simulink model "med_16a_2x_r113a"  (Simulink 7.4) (continued)
== system med_16a_2x_r113a/buffer1 == (continued)
      mask Help: eval('');xlDoc('-book','sysgen','-topic','Register');
      mask Display:
      | fprintf('','COMMENT: begin icon graphics');
      | patch([0 1 1 0 ],[0 0 1 1 ],[0.77 0.82 0.91]);
      | patch([0.25 0.1 0.316667 0.1 0.25 0.5 0.566667 0.633333 0.9 0.683333 0.483333 0.333333 0.533333 0.333333 0.483333 0.683333 0.9 0.633333 0.566667 0.5 0.25 ],[0.107143 0.267857 0.5 0.732143 0.892857 0.892857 0.821429 0.892857 0.892857 0.660714 0.875 0.714286 0.5 0.285714 0.125 0.339286 0.107143 0.107143 0.178571 0.107143 0.107143 ],[0.98 0.96 0.92]);
      | plot([0 1 1 0 0 ],[0 0 1 1 0 ]);
      | fprintf('','COMMENT: end icon graphics');
      | fprintf('','COMMENT: begin icon text');
      | color('black');port_label('input',1,'d');
      | color('black');port_label('input',2,'en');
      | color('black');port_label('output',1,'q');
      | color('black');disp('\bf{z^{-1}}','texmode','on');
      | fprintf('','COMMENT: end icon text');
  Register6 : S-Function  LoadFcn=xlBlockLoadCallback(gcbh);  FunctionName=sysgen  Parameters=ptable_  EnableBusSupport=off  mask(Xilinx Register Block){init=0, rst_sgadvanced=, rst=on, en_sgadvanced=, en=on, dbl_ovrd_sgadvanced=, dbl_ovrd=off, xl_use_area_sgadvanced=, xl_use_area=off, xl_area=[0,0,0,0,0,0,0], has_advanced_control=0, sggui_pos=21,47,436,219, block_type=register, block_version=11.4, sg_icon_stat=60,80,3,1,white,blue,0,b821322c,right,, sg_mask_display=|, sg_list_contents=, sg_blockgui_xml=}
      mask sg_mask_display=|
      | fprintf('','COMMENT: begin icon graphics');
      | patch([0 1 1 0 ],[0 0 1 1 ],[0.77 0.82 0.91]);
      | patch([0.233333 0.0666667 0.3 0.0666667 0.233333 0.5 0.566667 0.633333 0.916667 0.7 0.483333 0.333333 0.583333 0.333333 0.483333 0.7 0.916667 0.633333 0.566667 0.5 0.233333 ],[0.2 0.325 0.5 0.675 0.8 0.8 0.75 0.8 0.8 0.6375 0.8 0.6875 0.5 0.3125 0.2 0.3625 0.2 0.2 0.25 0.2 0.2 ],[0.98 0.96 0.92]);
      | plot([0 1 1 0 0 ],[0 0 1 1 0 ]);
      | fprintf('','COMMENT: end icon graphics');
      | fprintf('','COMMENT: begin icon text');
      | color('black');port_label('input',1,'d');
      | color('black');port_label('input',2,'rst');
      | color('black');port_label('input',3,'en');
      | color('black');port_label('output',1,'q');
      | color('black');disp('\bf{z^{-1}}','texmode','on');
      | fprintf('','COMMENT: end icon text');
      mask prompts: init "Initial value"; rst_sgadvanced ""; rst "Provide synchronous reset port"; en_sgadvanced ""; en "Provide enable port"; dbl_ovrd_sgadvanced ""; dbl_ovrd "Override with doubles"; xl_use_area_sgadvanced ""; xl_use_area "Define FPGA area for resource estimation"; xl_area "FPGA area [slices, FFs, BRAMs, LUTs, IOBs, emb. mults, TBUFs]"; has_advanced_control ""; sggui_pos ""; block_type ""; block_version ""; sg_icon_stat ""; sg_mask_display ""; sg_list_contents ""; sg_blockgui_xml ""
      mask help: Xilinx Register
      mask init: same as med_16a_2x_r113a/buffer1/Register2
      settings: CopyFcn=xlBlockMoveCallback(gcbh);  DeleteFcn=xlDestroyGui(gcbh);  ModelCloseFcn=xlDestroyGui(gcbh);  PreSaveFcn=xlBlockPreSaveCallback(gcbh);  PostSaveFcn=xlBlockPostSaveCallback(gcbh);  DestroyFcn=xlDestroyGui(gcbh);  OpenFcn=xlOpenGui(gcbh, 'register_gui.xml', @xlmagicenablement, -1)  CloseFcn=xlDestroyGui(gcbh);  MoveFcn=xlBlockMoveCallback(gcbh);
      mask Help: eval('');xlDoc('-book','sysgen','-topic','Register');
      mask Display:
      | fprintf('','COMMENT: begin icon graphics');
      | patch([0 1 1 0 ],[0 0 1 1 ],[0.77 0.82 0.91]);
      | patch([0.233333 0.0666667 0.3 0.0666667 0.233333 0.5 0.566667 0.633333 0.916667 0.7 0.483333 0.333333 0.583333 0.333333 0.483333 0.7 0.916667 0.633333 0.566667 0.5 0.233333 ],[0.2 0.325 0.5 0.675 0.8 0.8 0.75 0.8 0.8 0.6375 0.8 0.6875 0.5 0.3125 0.2 0.3625 0.2 0.2 0.25 0.2 0.2 ],[0.98 0.96 0.92]);
      | plot([0 1 1 0 0 ],[0 0 1 1 0 ]);
      | fprintf('','COMMENT: end icon graphics');
      | fprintf('','COMMENT: begin icon text');
      | color('black');port_label('input',1,'d');
      | color('black');port_label('input',2,'rst');
      | color('black');port_label('input',3,'en');
      | color('black');port_label('output',1,'q');
      | color('black');disp('\bf{z^{-1}}','texmode','on');
      | fprintf('','COMMENT: end icon text');
  Register7 : S-Function  LoadFcn=xlBlockLoadCallback(gcbh);  FunctionName=sysgen  Parameters=ptable_  EnableBusSupport=off  mask(Xilinx Register Block){init=0, rst_sgadvanced=, rst=on, en_sgadvanced=, en=on, dbl_ovrd_sgadvanced=, dbl_ovrd=off, xl_use_area_sgadvanced=, xl_use_area=off, xl_area=[0,0,0,0,0,0,0], has_advanced_control=0, sggui_pos=0,0,436,219, block_type=register, block_version=11.4, sg_icon_stat=60,74,3,1,white,blue,0,b821322c,right,, sg_mask_display=|, sg_list_contents=, sg_blockgui_xml=}
      mask sg_mask_display=|
      | fprintf('','COMMENT: begin icon graphics');
      | patch([0 1 1 0 ],[0 0 1 1 ],[0.77 0.82 0.91]);
      | patch([0.233333 0.0666667 0.3 0.0666667 0.233333 0.5 0.566667 0.633333 0.916667 0.7 0.483333 0.333333 0.583333 0.333333 0.483333 0.7 0.916667 0.633333 0.566667 0.5 0.233333 ],[0.175676 0.310811 0.5 0.689189 0.824324 0.824324 0.77027 0.824324 0.824324 0.648649 0.824324 0.702703 0.5 0.297297 0.175676 0.351351 0.175676 0.175676 0.22973 0.175676 0.175676 ],[0.98 0.96 0.92]);
      | plot([0 1 1 0 0 ],[0 0 1 1 0 ]);
      | fprintf('','COMMENT: end icon graphics');
      | fprintf('','COMMENT: begin icon text');
      | color('black');port_label('input',1,'d');
      | color('black');port_label('input',2,'rst');
      | color('black');port_label('input',3,'en');
      | color('black');port_label('output',1,'q');
      | color('black');disp('\bf{z^{-1}}','texmode','on');
      | fprintf('','COMMENT: end icon text');
      mask prompts: init "Initial value"; rst_sgadvanced ""; rst "Provide synchronous reset port"; en_sgadvanced ""; en "Provide enable port"; dbl_ovrd_sgadvanced ""; dbl_ovrd "Override with doubles"; xl_use_area_sgadvanced ""; xl_use_area "Define FPGA area for resource estimation"; xl_area "FPGA area [slices, FFs, BRAMs, LUTs, IOBs, emb. mults, TBUFs]"; has_advanced_control ""; sggui_pos ""; block_type ""; block_version ""; sg_icon_stat ""; sg_mask_display ""; sg_list_contents ""; sg_blockgui_xml ""
      mask help: Xilinx Register
      mask init: same as med_16a_2x_r113a/buffer1/Register2
      settings: CopyFcn=xlBlockMoveCallback(gcbh);  DeleteFcn=xlDestroyGui(gcbh);  ModelCloseFcn=xlDestroyGui(gcbh);  PreSaveFcn=xlBlockPreSaveCallback(gcbh);  PostSaveFcn=xlBlockPostSaveCallback(gcbh);  DestroyFcn=xlDestroyGui(gcbh);  OpenFcn=xlOpenGui(gcbh, 'register_gui.xml', @xlmagicenablement, -1)  CloseFcn=xlDestroyGui(gcbh);  MoveFcn=xlBlockMoveCallback(gcbh);
      mask Help: eval('');xlDoc('-book','sysgen','-topic','Register');
      mask Display:
      | fprintf('','COMMENT: begin icon graphics');
      | patch([0 1 1 0 ],[0 0 1 1 ],[0.77 0.82 0.91]);
      | patch([0.233333 0.0666667 0.3 0.0666667 0.233333 0.5 0.566667 0.633333 0.916667 0.7 0.483333 0.333333 0.583333 0.333333 0.483333 0.7 0.916667 0.633333 0.566667 0.5 0.233333 ],[0.175676 0.310811 0.5 0.689189 0.824324 0.824324 0.77027 0.824324 0.824324 0.648649 0.824324 0.702703 0.5 0.297297 0.175676 0.351351 0.175676 0.175676 0.22973 0.175676 0.175676 ],[0.98 0.96 0.92]);
      | plot([0 1 1 0 0 ],[0 0 1 1 0 ]);
      | fprintf('','COMMENT: end icon graphics');
      | fprintf('','COMMENT: begin icon text');
      | color('black');port_label('input',1,'d');
      | color('black');port_label('input',2,'rst');
      | color('black');port_label('input',3,'en');
      | color('black');port_label('output',1,'q');
      | color('black');disp('\bf{z^{-1}}','texmode','on');
      | fprintf('','COMMENT: end icon text');
  Relational1 : S-Function  LoadFcn=xlBlockLoadCallback(gcbh);  FunctionName=sysgen  Parameters=ptable_  EnableBusSupport=off  mask(Xilinx Arithmetic Relational Operator Block){mode_sgadvanced=, mode=a=b, en_sgadvanced=, en=off, latency=1, dbl_ovrd_sgadvanced=, dbl_ovrd=off, xl_use_area_sgadvanced=, xl_use_area=off, xl_area=[0, 0, 0, 0, 0, 0, 0], has_advanced_control=0, sggui_pos=21,72,436,219, block_type=relational, block_version=9.2.01, sg_icon_stat=55,51,2,1,white,blue,0,2d4703fd,right,, sg_mask_display=|, sg_list_contents={'table'=>{'mode'=>'popup(a=b|a!=b|a<b|a>b|a<=b|a>=b)','userSelections'=>{'mode'=>'a=b'}}}, sg_blockgui_xml=}
      mask sg_mask_display=|
      | fprintf('','COMMENT: begin icon graphics');
      | patch([0 1 1 0 ],[0 0 1 1 ],[0.77 0.82 0.91]);
      | patch([0.236364 0.0909091 0.309091 0.0909091 0.236364 0.472727 0.545455 0.618182 0.872727 0.672727 0.472727 0.327273 0.545455 0.327273 0.472727 0.672727 0.872727 0.618182 0.545455 0.472727 0.236364 ],[0.117647 0.27451 0.509804 0.745098 0.901961 0.901961 0.823529 0.901961 0.901961 0.686275 0.901961 0.745098 0.509804 0.27451 0.117647 0.333333 0.117647 0.117647 0.196078 0.117647 0.117647 ],[0.98 0.96 0.92]);
      | plot([0 1 1 0 0 ],[0 0 1 1 0 ]);
      | fprintf('','COMMENT: end icon graphics');
      | fprintf('','COMMENT: begin icon text');
      | color('black');port_label('input',1,'a');
      | color('black');port_label('input',2,'b');
      | color('black');disp('\newline\bf{a=b}\newlinez^{-1}','texmode','on');
      | fprintf('','COMMENT: end icon text');
      mask prompts: mode_sgadvanced ""; mode "Comparison:"; en_sgadvanced ""; en "Provide enable port"; dbl_ovrd_sgadvanced ""; dbl_ovrd "Override with doubles"; xl_use_area_sgadvanced ""; xl_use_area "Define FPGA area for resource estimation"; xl_area "FPGA area [slices, FFs, BRAMs, LUTs, IOBs, emb. mults, TBUFs]"; has_advanced_control ""; sggui_pos ""; block_type ""; block_version ""; sg_icon_stat ""; sg_mask_display ""; sg_list_contents ""; sg_blockgui_xml ""
      mask help: Xilinx Arithmetic Relational Operator
      mask init:
      | try
      |   tmp_gcb = gcb;
      |   tmp_gcbh = gcbh;
      |   if (strcmp('SysGenIndex',get_param(bdroot(tmp_gcbh),'tag')) && ~isempty(regexp(bdroot(tmp_gcb), '^xbs', 'once')))
      |     return;
      |   end;
      |   xlMungeMaskParams;
      |   block_config = 'sysgen_blockset:Relational_config';
      | 
      |   block_type='relational';
      | 
      |   serialized_declarations = '{''dbl_ovrd''=>''Int'',''en''=>''Int'',''latency''=>''Int'',''mode''=>''Int'',''block_type''=>''String''}';
      |   ptable_ = xlblockprep(get_param(tmp_gcb, 'MaskWSVariables'));
      | catch
      |   global dbgsysgen;
      |   if(~isempty(dbgsysgen) && dbgsysgen)
      |     e = regexprep(lasterr, '\n', '\nError: ');
      |     disp(['Error: While running MaskInit code on block ' tmp_gcb ': ' e]);
      |     error(e);
      |   end
      | end
      settings: CopyFcn=xlBlockMoveCallback(gcbh);  DeleteFcn=xlDestroyGui(gcbh);  ModelCloseFcn=xlDestroyGui(gcbh);  PreSaveFcn=xlBlockPreSaveCallback(gcbh);  PostSaveFcn=xlBlockPostSaveCallback(gcbh);  DestroyFcn=xlDestroyGui(gcbh);  OpenFcn=xlOpenGui(gcbh, 'relational_gui.xml', @xlmagicenablement, -1)  CloseFcn=xlDestroyGui(gcbh);  MoveFcn=xlBlockMoveCallback(gcbh);
      mask Help: eval('');xlDoc('-book','sysgen','-topic','Relational');
      mask StyleString: edit,popup(a=b|a!=b|a<b|a>b|a<=b|a>=b),edit,checkbox,edit,edit,checkbox,edit,checkbox,edit,edit,edit,edit,edit,edit,edit,edit,edit
      mask Display:
      | fprintf('','COMMENT: begin icon graphics');
      | patch([0 1 1 0 ],[0 0 1 1 ],[0.77 0.82 0.91]);
      | patch([0.236364 0.0909091 0.309091 0.0909091 0.236364 0.472727 0.545455 0.618182 0.872727 0.672727 0.472727 0.327273 0.545455 0.327273 0.472727 0.672727 0.872727 0.618182 0.545455 0.472727 0.236364 ],[0.117647 0.27451 0.509804 0.745098 0.901961 0.901961 0.823529 0.901961 0.901961 0.686275 0.901961 0.745098 0.509804 0.27451 0.117647 0.333333 0.117647 0.117647 0.196078 0.117647 0.117647 ],[0.98 0.96 0.92]);
      | plot([0 1 1 0 0 ],[0 0 1 1 0 ]);
      | fprintf('','COMMENT: end icon graphics');
      | fprintf('','COMMENT: begin icon text');
      | color('black');port_label('input',1,'a');
      | color('black');port_label('input',2,'b');
      | color('black');disp('\newline\bf{a=b}\newlinez^{-1}','texmode','on');
      | fprintf('','COMMENT: end icon text');
  Relational3 : S-Function  LoadFcn=xlBlockLoadCallback(gcbh);  FunctionName=sysgen  Parameters=ptable_  EnableBusSupport=off  mask(Xilinx Arithmetic Relational Operator Block){mode_sgadvanced=, mode=a=b, en_sgadvanced=, en=off, latency=1, dbl_ovrd_sgadvanced=, dbl_ovrd=off, xl_use_area_sgadvanced=, xl_use_area=off, xl_area=[0, 0, 0, 0, 0, 0, 0], has_advanced_control=0, sggui_pos=21,72,436,219, block_type=relational, block_version=9.2.01, sg_icon_stat=55,51,2,1,white,blue,0,2d4703fd,right,, sg_mask_display=|, sg_list_contents={'table'=>{'mode'=>'popup(a=b|a!=b|a<b|a>b|a<=b|a>=b)','userSelections'=>{'mode'=>'a=b'}}}, sg_blockgui_xml=}
      mask sg_mask_display=|
      | fprintf('','COMMENT: begin icon graphics');
      | patch([0 1 1 0 ],[0 0 1 1 ],[0.77 0.82 0.91]);
      | patch([0.236364 0.0909091 0.309091 0.0909091 0.236364 0.472727 0.545455 0.618182 0.872727 0.672727 0.472727 0.327273 0.545455 0.327273 0.472727 0.672727 0.872727 0.618182 0.545455 0.472727 0.236364 ],[0.117647 0.27451 0.509804 0.745098 0.901961 0.901961 0.823529 0.901961 0.901961 0.686275 0.901961 0.745098 0.509804 0.27451 0.117647 0.333333 0.117647 0.117647 0.196078 0.117647 0.117647 ],[0.98 0.96 0.92]);
      | plot([0 1 1 0 0 ],[0 0 1 1 0 ]);
      | fprintf('','COMMENT: end icon graphics');
      | fprintf('','COMMENT: begin icon text');
      | color('black');port_label('input',1,'a');
      | color('black');port_label('input',2,'b');
      | color('black');disp('\newline\bf{a=b}\newlinez^{-1}','texmode','on');
      | fprintf('','COMMENT: end icon text');
      mask prompts: mode_sgadvanced ""; mode "Comparison:"; en_sgadvanced ""; en "Provide enable port"; dbl_ovrd_sgadvanced ""; dbl_ovrd "Override with doubles"; xl_use_area_sgadvanced ""; xl_use_area "Define FPGA area for resource estimation"; xl_area "FPGA area [slices, FFs, BRAMs, LUTs, IOBs, emb. mults, TBUFs]"; has_advanced_control ""; sggui_pos ""; block_type ""; block_version ""; sg_icon_stat ""; sg_mask_display ""; sg_list_contents ""; sg_blockgui_xml ""
      mask help: Xilinx Arithmetic Relational Operator
      mask init: same as med_16a_2x_r113a/buffer1/Relational1
      settings: CopyFcn=xlBlockMoveCallback(gcbh);  DeleteFcn=xlDestroyGui(gcbh);  ModelCloseFcn=xlDestroyGui(gcbh);  PreSaveFcn=xlBlockPreSaveCallback(gcbh);  PostSaveFcn=xlBlockPostSaveCallback(gcbh);  DestroyFcn=xlDestroyGui(gcbh);  OpenFcn=xlOpenGui(gcbh, 'relational_gui.xml', @xlmagicenablement, -1)  CloseFcn=xlDestroyGui(gcbh);  MoveFcn=xlBlockMoveCallback(gcbh);
      mask Help: eval('');xlDoc('-book','sysgen','-topic','Relational');
      mask StyleString: edit,popup(a=b|a!=b|a<b|a>b|a<=b|a>=b),edit,checkbox,edit,edit,checkbox,edit,checkbox,edit,edit,edit,edit,edit,edit,edit,edit,edit
      mask Display:
      | fprintf('','COMMENT: begin icon graphics');
      | patch([0 1 1 0 ],[0 0 1 1 ],[0.77 0.82 0.91]);
      | patch([0.236364 0.0909091 0.309091 0.0909091 0.236364 0.472727 0.545455 0.618182 0.872727 0.672727 0.472727 0.327273 0.545455 0.327273 0.472727 0.672727 0.872727 0.618182 0.545455 0.472727 0.236364 ],[0.117647 0.27451 0.509804 0.745098 0.901961 0.901961 0.823529 0.901961 0.901961 0.686275 0.901961 0.745098 0.509804 0.27451 0.117647 0.333333 0.117647 0.117647 0.196078 0.117647 0.117647 ],[0.98 0.96 0.92]);
      | plot([0 1 1 0 0 ],[0 0 1 1 0 ]);
      | fprintf('','COMMENT: end icon graphics');
      | fprintf('','COMMENT: begin icon text');
      | color('black');port_label('input',1,'a');
      | color('black');port_label('input',2,'b');
      | color('black');disp('\newline\bf{a=b}\newlinez^{-1}','texmode','on');
      | fprintf('','COMMENT: end icon text');
  Slice3 : S-Function  LoadFcn=xlBlockLoadCallback(gcbh);  FunctionName=sysgen  Parameters=ptable_  EnableBusSupport=off  mask(Xilinx Bit Slice Extractor Block){infoedit=Extracts a given range of bits from each input sample and presents it at the output.  The output type is ordinarily unsigned with binary point at zero, but can be Boolean when the slice is one bit wide.<br><br>Hardware notes: In hardware this block costs nothing., nbits=16, boolean_output_sgadvanced=, boolean_output=off, mode_sgadvanced=, mode=Lower Bit Location + Width, bit1=0, base1_sgadvanced=, base1=MSB of Input, bit0=0, base0_sgadvanced=, base0=LSB of Input, dbl_ovrd_sgadvanced=, dbl_ovrd=off, has_advanced_control=0, sggui_pos=22,74,631,621, block_type=slice, block_version=11.4, sg_icon_stat=60,20,1,1,white,blue,0,1fd851a7,right,, sg_mask_display=|, sg_list_contents=, sg_blockgui_xml=}
      mask sg_mask_display=|
      | fprintf('','COMMENT: begin icon graphics');
      | patch([0 1 1 0 ],[0 0 1 1 ],[0.77 0.82 0.91]);
      | patch([0.4 0.35 0.433333 0.35 0.4 0.483333 0.5 0.516667 0.616667 0.55 0.483333 0.433333 0.516667 0.433333 0.483333 0.55 0.616667 0.516667 0.5 0.483333 0.4 ],[0.1 0.25 0.5 0.75 0.9 0.9 0.85 0.9 0.9 0.7 0.9 0.75 0.5 0.25 0.1 0.3 0.1 0.1 0.15 0.1 0.1 ],[0.98 0.96 0.92]);
      | plot([0 1 1 0 0 ],[0 0 1 1 0 ]);
      | fprintf('','COMMENT: end icon graphics');
      | fprintf('','COMMENT: begin icon text');
      | color('black');port_label('output',1,'[a:b]');
      | fprintf('','COMMENT: end icon text');
      mask prompts: infoedit ""; nbits "Width of slice (number of bits)"; boolean_output_sgadvanced ""; boolean_output "Boolean output"; mode_sgadvanced ""; mode "Specify range as"; bit1 "Offset of top bit"; base1_sgadvanced ""; base1 "Relative to"; bit0 "Offset of bottom bit"; base0_sgadvanced ""; base0 "Relative to"; dbl_ovrd_sgadvanced ""; dbl_ovrd "Override with doubles"; has_advanced_control ""; sggui_pos ""; block_type ""; block_version ""; sg_icon_stat ""; sg_mask_display ""; sg_list_contents ""; sg_blockgui_xml ""
      mask help:
      | Extracts a given range of bits from each input sample and presents it at the output.  The output type is ordinarily unsigned with binary point at zero, but can be Boolean when the slice is one bit wide.
      |
      | Hardware notes: In hardware this block costs nothing.
      mask init:
      | try
      |   tmp_gcb = gcb;
      |   tmp_gcbh = gcbh;
      |   if (strcmp('SysGenIndex',get_param(bdroot(tmp_gcbh),'tag')) && ~isempty(regexp(bdroot(tmp_gcb), '^xbs', 'once')))
      |     return;
      |   end;
      |   xlMungeMaskParams;
      |   block_config = 'sysgen_blockset:Slice_config';
      | 
      |   block_type='slice';
      | 
      |   serialized_declarations = '{''base0''=>''Int'',''base1''=>''Int'',''bit0''=>''Int'',''bit1''=>''Int'',''boolean_output''=>''Int'',''mode''=>''Int'',''nbits''=>''Int'',''sim_engine''=>''Int'',''sysgen_root''=>''String'',''block_type''=>''String''}';
      |   xlslice_init();
      |   ptable_ = xlblockprep(get_param(tmp_gcb, 'MaskWSVariables'));
      | catch
      |   global dbgsysgen;
      |   if(~isempty(dbgsysgen) && dbgsysgen)
      |     e = regexprep(lasterr, '\n', '\nError: ');
      |     disp(['Error: While running MaskInit code on block ' tmp_gcb ': ' e]);
      |     error(e);
      |   end
      | end
      settings: CopyFcn=xlBlockMoveCallback(gcbh);  DeleteFcn=xlDestroyGui(gcbh);  ModelCloseFcn=xlDestroyGui(gcbh);  PreSaveFcn=xlBlockPreSaveCallback(gcbh);  PostSaveFcn=xlBlockPostSaveCallback(gcbh);  DestroyFcn=xlDestroyGui(gcbh);  OpenFcn=xlOpenGui(gcbh, 'slice_gui.xml', @sliceenablement, -1)  CloseFcn=xlDestroyGui(gcbh);  MoveFcn=xlBlockMoveCallback(gcbh);
      mask Help: eval('');xlDoc('-book','sysgen','-topic','Slice');
      mask StyleString: edit,edit,edit,checkbox,edit,popup(Two Bit Locations|Upper Bit Location + Width|Lower Bit Location + Width),edit,edit,popup(LSB of Input|Binary Point of Input|MSB of Input),edit,edit,popup(LSB of Input|Binary Point of Input|MSB of Input),edit,checkbox,edit,edit,edit,edit,edit,edit,edit,edit
      mask Display:
      | fprintf('','COMMENT: begin icon graphics');
      | patch([0 1 1 0 ],[0 0 1 1 ],[0.77 0.82 0.91]);
      | patch([0.4 0.35 0.433333 0.35 0.4 0.483333 0.5 0.516667 0.616667 0.55 0.483333 0.433333 0.516667 0.433333 0.483333 0.55 0.616667 0.516667 0.5 0.483333 0.4 ],[0.1 0.25 0.5 0.75 0.9 0.9 0.85 0.9 0.9 0.7 0.9 0.75 0.5 0.25 0.1 0.3 0.1 0.1 0.15 0.1 0.1 ],[0.98 0.96 0.92]);
      | plot([0 1 1 0 0 ],[0 0 1 1 0 ]);
      | fprintf('','COMMENT: end icon graphics');
      | fprintf('','COMMENT: begin icon text');
      | color('black');port_label('output',1,'[a:b]');
      | fprintf('','COMMENT: end icon text');
  Slice4 : S-Function  LoadFcn=xlBlockLoadCallback(gcbh);  FunctionName=sysgen  Parameters=ptable_  EnableBusSupport=off  mask(Xilinx Bit Slice Extractor Block){infoedit=Extracts a given range of bits from each input sample and presents it at the output.  The output type is ordinarily unsigned with binary point at zero, but can be Boolean when the slice is one bit wide.<br><br>Hardware notes: In hardware this block costs nothing., nbits=1, boolean_output_sgadvanced=, boolean_output=on, mode_sgadvanced=, mode=Lower Bit Location + Width, bit1=0, base1_sgadvanced=, base1=MSB of Input, bit0=16, base0_sgadvanced=, base0=LSB of Input, dbl_ovrd_sgadvanced=, dbl_ovrd=off, has_advanced_control=0, sggui_pos=22,74,631,621, block_type=slice, block_version=11.4, sg_icon_stat=60,20,1,1,white,blue,0,1fd851a7,right,, sg_mask_display=|, sg_list_contents=, sg_blockgui_xml=}
      mask sg_mask_display=|
      | fprintf('','COMMENT: begin icon graphics');
      | patch([0 1 1 0 ],[0 0 1 1 ],[0.77 0.82 0.91]);
      | patch([0.4 0.35 0.433333 0.35 0.4 0.483333 0.5 0.516667 0.616667 0.55 0.483333 0.433333 0.516667 0.433333 0.483333 0.55 0.616667 0.516667 0.5 0.483333 0.4 ],[0.1 0.25 0.5 0.75 0.9 0.9 0.85 0.9 0.9 0.7 0.9 0.75 0.5 0.25 0.1 0.3 0.1 0.1 0.15 0.1 0.1 ],[0.98 0.96 0.92]);
      | plot([0 1 1 0 0 ],[0 0 1 1 0 ]);
      | fprintf('','COMMENT: end icon graphics');
      | fprintf('','COMMENT: begin icon text');
      | color('black');port_label('output',1,'[a:b]');
      | fprintf('','COMMENT: end icon text');
      mask prompts: infoedit ""; nbits "Width of slice (number of bits)"; boolean_output_sgadvanced ""; boolean_output "Boolean output"; mode_sgadvanced ""; mode "Specify range as"; bit1 "Offset of top bit"; base1_sgadvanced ""; base1 "Relative to"; bit0 "Offset of bottom bit"; base0_sgadvanced ""; base0 "Relative to"; dbl_ovrd_sgadvanced ""; dbl_ovrd "Override with doubles"; has_advanced_control ""; sggui_pos ""; block_type ""; block_version ""; sg_icon_stat ""; sg_mask_display ""; sg_list_contents ""; sg_blockgui_xml ""
      mask help:
      | Extracts a given range of bits from each input sample and presents it at the output.  The output type is ordinarily unsigned with binary point at zero, but can be Boolean when the slice is one bit wide.
      |
      | Hardware notes: In hardware this block costs nothing.
      mask init: same as med_16a_2x_r113a/buffer1/Slice3
      settings: CopyFcn=xlBlockMoveCallback(gcbh);  DeleteFcn=xlDestroyGui(gcbh);  ModelCloseFcn=xlDestroyGui(gcbh);  PreSaveFcn=xlBlockPreSaveCallback(gcbh);  PostSaveFcn=xlBlockPostSaveCallback(gcbh);  DestroyFcn=xlDestroyGui(gcbh);  OpenFcn=xlOpenGui(gcbh, 'slice_gui.xml', @sliceenablement, -1)  CloseFcn=xlDestroyGui(gcbh);  MoveFcn=xlBlockMoveCallback(gcbh);
      mask Help: eval('');xlDoc('-book','sysgen','-topic','Slice');
      mask StyleString: edit,edit,edit,checkbox,edit,popup(Two Bit Locations|Upper Bit Location + Width|Lower Bit Location + Width),edit,edit,popup(LSB of Input|Binary Point of Input|MSB of Input),edit,edit,popup(LSB of Input|Binary Point of Input|MSB of Input),edit,checkbox,edit,edit,edit,edit,edit,edit,edit,edit
      mask Display:
      | fprintf('','COMMENT: begin icon graphics');
      | patch([0 1 1 0 ],[0 0 1 1 ],[0.77 0.82 0.91]);
      | patch([0.4 0.35 0.433333 0.35 0.4 0.483333 0.5 0.516667 0.616667 0.55 0.483333 0.433333 0.516667 0.433333 0.483333 0.55 0.616667 0.516667 0.5 0.483333 0.4 ],[0.1 0.25 0.5 0.75 0.9 0.9 0.85 0.9 0.9 0.7 0.9 0.75 0.5 0.25 0.1 0.3 0.1 0.1 0.15 0.1 0.1 ],[0.98 0.96 0.92]);
      | plot([0 1 1 0 0 ],[0 0 1 1 0 ]);
      | fprintf('','COMMENT: end icon graphics');
      | fprintf('','COMMENT: begin icon text');
      | color('black');port_label('output',1,'[a:b]');
      | fprintf('','COMMENT: end icon text');
  Slice5 : S-Function  LoadFcn=xlBlockLoadCallback(gcbh);  FunctionName=sysgen  Parameters=ptable_  EnableBusSupport=off  mask(Xilinx Bit Slice Extractor Block){infoedit=Extracts a given range of bits from each input sample and presents it at the output.  The output type is ordinarily unsigned with binary point at zero, but can be Boolean when the slice is one bit wide.<br><br>Hardware notes: In hardware this block costs nothing., nbits=1, boolean_output_sgadvanced=, boolean_output=on, mode_sgadvanced=, mode=Lower Bit Location + Width, bit1=0, base1_sgadvanced=, base1=MSB of Input, bit0=log2(N_xeng), base0_sgadvanced=, base0=LSB of Input, dbl_ovrd_sgadvanced=, dbl_ovrd=off, has_advanced_control=0, sggui_pos=641,74,631,621, block_type=slice, block_version=11.4, sg_icon_stat=30,18,1,1,white,blue,0,1fd851a7,right,, sg_mask_display=|, sg_list_contents=, sg_blockgui_xml=}
      mask sg_mask_display=|
      | fprintf('','COMMENT: begin icon graphics');
      | patch([0 1 1 0 ],[0 0 1 1 ],[0.77 0.82 0.91]);
      | patch([0.333333 0.233333 0.366667 0.233333 0.333333 0.5 0.533333 0.566667 0.733333 0.6 0.466667 0.366667 0.5 0.366667 0.466667 0.6 0.733333 0.566667 0.533333 0.5 0.333333 ],[0.111111 0.277778 0.5 0.722222 0.888889 0.888889 0.833333 0.888889 0.888889 0.666667 0.888889 0.722222 0.5 0.277778 0.111111 0.333333 0.111111 0.111111 0.166667 0.111111 0.111111 ],[0.98 0.96 0.92]);
      | plot([0 1 1 0 0 ],[0 0 1 1 0 ]);
      | fprintf('','COMMENT: end icon graphics');
      | fprintf('','COMMENT: begin icon text');
      | color('black');port_label('output',1,'[a:b]');
      | fprintf('','COMMENT: end icon text');
      mask prompts: infoedit ""; nbits "Width of slice (number of bits)"; boolean_output_sgadvanced ""; boolean_output "Boolean output"; mode_sgadvanced ""; mode "Specify range as"; bit1 "Offset of top bit"; base1_sgadvanced ""; base1 "Relative to"; bit0 "Offset of bottom bit"; base0_sgadvanced ""; base0 "Relative to"; dbl_ovrd_sgadvanced ""; dbl_ovrd "Override with doubles"; has_advanced_control ""; sggui_pos ""; block_type ""; block_version ""; sg_icon_stat ""; sg_mask_display ""; sg_list_contents ""; sg_blockgui_xml ""
      mask help:
      | Extracts a given range of bits from each input sample and presents it at the output.  The output type is ordinarily unsigned with binary point at zero, but can be Boolean when the slice is one bit wide.
      |
      | Hardware notes: In hardware this block costs nothing.
      mask init: same as med_16a_2x_r113a/buffer1/Slice3
      settings: CopyFcn=xlBlockMoveCallback(gcbh);  DeleteFcn=xlDestroyGui(gcbh);  ModelCloseFcn=xlDestroyGui(gcbh);  PreSaveFcn=xlBlockPreSaveCallback(gcbh);  PostSaveFcn=xlBlockPostSaveCallback(gcbh);  DestroyFcn=xlDestroyGui(gcbh);  OpenFcn=xlOpenGui(gcbh, 'slice_gui.xml', @sliceenablement, -1)  CloseFcn=xlDestroyGui(gcbh);  MoveFcn=xlBlockMoveCallback(gcbh);
      mask Help: eval('');xlDoc('-book','sysgen','-topic','Slice');
      mask StyleString: edit,edit,edit,checkbox,edit,popup(Two Bit Locations|Upper Bit Location + Width|Lower Bit Location + Width),edit,edit,popup(LSB of Input|Binary Point of Input|MSB of Input),edit,edit,popup(LSB of Input|Binary Point of Input|MSB of Input),edit,checkbox,edit,edit,edit,edit,edit,edit,edit,edit
      mask Display:
      | fprintf('','COMMENT: begin icon graphics');
      | patch([0 1 1 0 ],[0 0 1 1 ],[0.77 0.82 0.91]);
      | patch([0.333333 0.233333 0.366667 0.233333 0.333333 0.5 0.533333 0.566667 0.733333 0.6 0.466667 0.366667 0.5 0.366667 0.466667 0.6 0.733333 0.566667 0.533333 0.5 0.333333 ],[0.111111 0.277778 0.5 0.722222 0.888889 0.888889 0.833333 0.888889 0.888889 0.666667 0.888889 0.722222 0.5 0.277778 0.111111 0.333333 0.111111 0.111111 0.166667 0.111111 0.111111 ],[0.98 0.96 0.92]);
      | plot([0 1 1 0 0 ],[0 0 1 1 0 ]);
      | fprintf('','COMMENT: end icon graphics');
      | fprintf('','COMMENT: begin icon text');
      | color('black');port_label('output',1,'[a:b]');
      | fprintf('','COMMENT: end icon text');
  end_window_detect : Subsystem
  pipeline2 : Subsystem  mask(pipeline){latency=1}
      mask help:
      | An explicitly laid-out delay line to avoid mapping to SRL16s
      | for use in pipelining to help achieve timing closure.
      mask init:
      | pipeline_init(gcb, 'latency', latency);
      mask Display:
      | port_label('input', 1, 'd');
      | port_label('output', 1, 'q');
      | disp(['Z^-', num2str(latency)]);
  pipeline3 : Subsystem  mask(pipeline){latency=1}
      mask help:
      | An explicitly laid-out delay line to avoid mapping to SRL16s
      | for use in pipelining to help achieve timing closure.
      mask init:
      | pipeline_init(gcb, 'latency', latency);
      mask Display:
      | port_label('input', 1, 'd');
      | port_label('output', 1, 'q');
      | disp(['Z^-', num2str(latency)]);
  pulse_ext : Subsystem  mask(pulse_ext){pulse_len=(2^N_int) * (N_ants)}
      mask prompts: pulse_len "Length of Pulse"
      mask help:
      | Extends a boolean signal to be high for the specified number of clocks after
      | the last high input.
      mask init:
      | bits = ceil(log2(pulse_len+1));
      mask Help: eval('xlWeb(''http://casper.berkeley.edu/wiki/Pulse_ext'')')
  pulse_ext1 : Subsystem  mask(pulse_ext){pulse_len=(2^N_int) * (N_ants)}
      mask prompts: pulse_len "Length of Pulse"
      mask help:
      | Extends a boolean signal to be high for the specified number of clocks after
      | the last high input.
      mask init:
      | bits = ceil(log2(pulse_len+1));
      mask Help: eval('xlWeb(''http://casper.berkeley.edu/wiki/Pulse_ext'')')
  pulse_ext2 : Subsystem  mask(pulse_ext){pulse_len=(2^N_int) * (N_ants)}
      mask prompts: pulse_len "Length of Pulse"
      mask help:
      | Extends a boolean signal to be high for the specified number of clocks after
      | the last high input.
      mask init:
      | bits = ceil(log2(pulse_len+1));
      mask Help: eval('xlWeb(''http://casper.berkeley.edu/wiki/Pulse_ext'')')
  pulse_ext3 : Subsystem  mask(pulse_ext){pulse_len=(2^N_int) * (N_ants)}
      mask prompts: pulse_len "Length of Pulse"
      mask help:
      | Extends a boolean signal to be high for the specified number of clocks after
      | the last high input.
      mask init:
      | bits = ceil(log2(pulse_len+1));
      mask Help: eval('xlWeb(''http://casper.berkeley.edu/wiki/Pulse_ext'')')
  pulse_ext4 : Subsystem  mask(pulse_ext){pulse_len=(2^N_int) * (N_ants)}
      mask prompts: pulse_len "Length of Pulse"
      mask help:
      | Extends a boolean signal to be high for the specified number of clocks after
      | the last high input.
      mask init:
      | bits = ceil(log2(pulse_len+1));
      mask Help: eval('xlWeb(''http://casper.berkeley.edu/wiki/Pulse_ext'')')
  rx_buf : S-Function  LoadFcn=xlBlockLoadCallback(gcbh);  FunctionName=sysgen  Parameters=ptable_  EnableBusSupport=off  mask(Xilinx Dual Port Random Access Memory Block){depth=2*2^ceil(log2((2^N_int) * (N_ants))), initVector=0, distributed_mem_sgadvanced=, distributed_mem=Block RAM, init_a=0, init_b=0, rst_a_sgadvanced=, rst_a=off, rst_b_sgadvanced=, rst_b=off, en_a_sgadvanced=, en_a=off, en_b_sgadvanced=, en_b=off, latency=2, write_mode_A_sgadvanced=, write_mode_A=Read Before Write, write_mode_B_sgadvanced=, write_mode_B=Read Before Write, dbl_ovrd_sgadvanced=, dbl_ovrd=off, optimize_sgadvanced=, optimize=Area, use_rpm_sgadvanced=, use_rpm=on, xl_use_area_sgadvanced=, xl_use_area=off, xl_area=[0,0,0,0,0,0,0], explicit_period=, period=, infoedit=, has_advanced_control=0, sggui_pos=0,0,460,478, block_type=dpram, block_version=11.4, sg_icon_stat=130,579,6,2,white,blue,0,28af736d,right,, sg_mask_display=|, sg_list_contents=, sg_blockgui_xml=}
      mask sg_mask_display=|
      | fprintf('','COMMENT: begin icon graphics');
      | patch([0 1 1 0 ],[0 0 1 1 ],[0.77 0.82 0.91]);
      | patch([0.238462 0.0692308 0.3 0.0692308 0.238462 0.5 0.569231 0.638462 0.923077 0.7 0.484615 0.330769 0.569231 0.330769 0.484615 0.7 0.923077 0.638462 0.569231 0.5 0.238462 ],[0.412781 0.450777 0.502591 0.554404 0.592401 0.592401 0.576857 0.592401 0.592401 0.542314 0.590674 0.556131 0.502591 0.44905 0.414508 0.462867 0.412781 0.412781 0.428325 0.412781 0.412781 ],[0.98 0.96 0.92]);
      | plot([0 1 1 0 0 ],[0 0 1 1 0 ]);
      | fprintf('','COMMENT: end icon graphics');
      | fprintf('','COMMENT: begin icon text');
      | color('black');port_label('input',1,'addra');
      | color('black');port_label('input',2,'dina');
      | color('black');port_label('input',3,'wea');
      | color('black');port_label('input',4,'addrb');
      | color('black');port_label('input',5,'dinb');
      | color('black');port_label('input',6,'web');
      | color('black');port_label('output',1,'A');
      | color('black');port_label('output',2,'B');
      | fprintf('','COMMENT: end icon text');
      mask prompts: initVector "Initial value vector"; distributed_mem_sgadvanced ""; distributed_mem "Memory Type"; init_a "Initial value for port <tt>A</tt> output register"; init_b "Initial value for port <tt>B</tt> output register"; rst_a_sgadvanced ""; rst_a "Provide synchronous reset port for port <tt>A</tt> output register"; rst_b_sgadvanced ""; rst_b "Provide synchronous reset port for port <tt>B</tt> output register"; en_a_sgadvanced ""; en_a "Provide enable port for port <tt>A</tt>"; en_b_sgadvanced ""; en_b "Provide enable port for port <tt>B</tt>"; write_mode_A_sgadvanced ""; write_mode_A "Port <tt>A</tt>"; write_mode_B_sgadvanced ""; write_mode_B "Port <tt>B</tt>"; dbl_ovrd_sgadvanced ""; dbl_ovrd "Override with doubles"; optimize_sgadvanced ""; optimize "Optimize for"; use_rpm_sgadvanced ""; use_rpm "Use core placement information"; xl_use_area_sgadvanced ""; xl_use_area "Define FPGA area for resource estimation"; xl_area "FPGA area [slices, FFs, BRAMs, LUTs, IOBs, emb. mults, TBUFs]"; explicit_period ""; period ""; infoedit ""; has_advanced_control ""; sggui_pos ""; block_type ""; block_version ""; sg_icon_stat ""; sg_mask_display ""; sg_list_contents ""; sg_blockgui_xml ""
      mask help: Xilinx Dual Port Random Access Memory
      mask init:
      | try
      |   tmp_gcb = gcb;
      |   tmp_gcbh = gcbh;
      |   if (strcmp('SysGenIndex',get_param(bdroot(tmp_gcbh),'tag')) && ~isempty(regexp(bdroot(tmp_gcb), '^xbs', 'once')))
      |     return;
      |   end;
      |   xlMungeMaskParams;
      |   block_config = 'sysgen_blockset:Dpram_config';
      | 
      |   block_type='dpram';
      | 
      |   serialized_declarations = '{''dblOvrd''=>''Int'',''depth''=>''Int'',''distributed_mem''=>''Int'',''en_a''=>''Int'',''en_b''=>''Int'',''init''=>''DoubleVector'',''initVector''=>''DoubleVector'',''init_a''=>''Double'',''init_b''=>''Double'',''latency''=>''Int'',''optimize''=>''Int'',''rst_a''=>''Int'',''rst_b''=>''Int'',''sim_engine''=>''Int'',''use_rpm''=>''Int'',''write_mode_A''=>''Int'',''write_mode_B''=>''Int'',''write_mode_a''=>''Int'',''write_mode_b''=>''Int'',''block_type''=>''String''}';
      |   xldpram_init();
      |   ptable_ = xlblockprep(get_param(tmp_gcb, 'MaskWSVariables'));
      | catch
      |   global dbgsysgen;
      |   if(~isempty(dbgsysgen) && dbgsysgen)
      |     e = regexprep(lasterr, '\n', '\nError: ');
      |     disp(['Error: While running MaskInit code on block ' tmp_gcb ': ' e]);
      |     error(e);
      |   end
      | end
      settings: CopyFcn=xlBlockMoveCallback(gcbh);  DeleteFcn=xlDestroyGui(gcbh);  ModelCloseFcn=xlDestroyGui(gcbh);  PreSaveFcn=xlBlockPreSaveCallback(gcbh);  PostSaveFcn=xlBlockPostSaveCallback(gcbh);  DestroyFcn=xlDestroyGui(gcbh);  OpenFcn=xlOpenGui(gcbh, 'dpram_gui.xml', @dpramenablement, -1)  CloseFcn=xlDestroyGui(gcbh);  MoveFcn=xlBlockMoveCallback(gcbh);
      mask Help: eval('');xlDoc('-book','sysgen','-topic','Dual_Port_RAM');
      mask StyleString: edit,edit,edit,popup(Distributed memory|Block RAM),edit,edit,edit,checkbox,edit,checkbox,edit,checkbox,edit,checkbox,edit,edit,popup(Read After Write|Read Before Write|No Read On Write),edit,popup(Read After Write|Read Before Write|No Read On Write),edit,checkbox,edit,popup(Area|Speed),edit,checkbox,edit,checkbox,edit,edit,edit,edit,edit,edit,edit,edit,edit,edit,edit,edit
      mask Display:
      | fprintf('','COMMENT: begin icon graphics');
      | patch([0 1 1 0 ],[0 0 1 1 ],[0.77 0.82 0.91]);
      | patch([0.238462 0.0692308 0.3 0.0692308 0.238462 0.5 0.569231 0.638462 0.923077 0.7 0.484615 0.330769 0.569231 0.330769 0.484615 0.7 0.923077 0.638462 0.569231 0.5 0.238462 ],[0.412781 0.450777 0.502591 0.554404 0.592401 0.592401 0.576857 0.592401 0.592401 0.542314 0.590674 0.556131 0.502591 0.44905 0.414508 0.462867 0.412781 0.412781 0.428325 0.412781 0.412781 ],[0.98 0.96 0.92]);
      | plot([0 1 1 0 0 ],[0 0 1 1 0 ]);
      | fprintf('','COMMENT: end icon graphics');
      | fprintf('','COMMENT: begin icon text');
      | color('black');port_label('input',1,'addra');
      | color('black');port_label('input',2,'dina');
      | color('black');port_label('input',3,'wea');
      | color('black');port_label('input',4,'addrb');
      | color('black');port_label('input',5,'dinb');
      | color('black');port_label('input',6,'web');
      | color('black');port_label('output',1,'A');
      | color('black');port_label('output',2,'B');
      | fprintf('','COMMENT: end icon text');
  window_position : S-Function  LoadFcn=xlBlockLoadCallback(gcbh);  FunctionName=sysgen  Parameters=ptable_  EnableBusSupport=off  mask(Xilinx Counter Block){infoedit=Hardware notes: Free running counters are the least expensive in hardware.  A count limited counter is implemented by combining a counter with a comparator., cnt_type_sgadvanced=, cnt_type=Count Limited, cnt_to=((2^N_int) * (N_ants))/4-1, operation_sgadvanced=, operation=Up, start_count=0, cnt_by_val=1, arith_type_sgadvanced=, arith_type=Unsigned, n_bits=ceil(log2(((2^N_int) * (N_ants))/4-1)), bin_pt=0, load_pin_sgadvanced=, load_pin=off, rst_sgadvanced=, rst=on, en_sgadvanced=, en=on, explicit_period_sgadvanced=, explicit_period=off, period=1, dbl_ovrd_sgadvanced=, dbl_ovrd=off, use_behavioral_HDL_sgadvanced=, use_behavioral_HDL=off, implementation_sgadvanced=, implementation=Fabric, xl_use_area_sgadvanced=, xl_use_area=off, xl_area=[0, 0, 0, 0, 0, 0, 0], use_rpm=on, xlcconfig=, has_advanced_control=0, sggui_pos=38,-20,1038,822, block_type=counter, block_version=9.2.01, sg_icon_stat=80,182,2,1,white,blue,0,d605779c,right,, sg_mask_display=|, sg_list_contents=, sg_blockgui_xml=}
      mask sg_mask_display=|
      | fprintf('','COMMENT: begin icon graphics');
      | patch([0 1 1 0 ],[0 0 1 1 ],[0.77 0.82 0.91]);
      | patch([0.225 0.0625 0.3 0.0625 0.225 0.4875 0.5625 0.6375 0.925 0.7 0.4875 0.3375 0.575 0.3375 0.4875 0.7 0.925 0.6375 0.5625 0.4875 0.225 ],[0.32967 0.401099 0.505495 0.60989 0.681319 0.681319 0.648352 0.681319 0.681319 0.582418 0.675824 0.60989 0.505495 0.401099 0.335165 0.428571 0.32967 0.32967 0.362637 0.32967 0.32967 ],[0.98 0.96 0.92]);
      | plot([0 1 1 0 0 ],[0 0 1 1 0 ]);
      | fprintf('','COMMENT: end icon graphics');
      | fprintf('','COMMENT: begin icon text');
      | color('black');port_label('input',1,'rst');
      | color('black');port_label('input',2,'en');
      | color('black');port_label('output',1,'out');
      | fprintf('','COMMENT: end icon text');
      mask prompts: infoedit ""; cnt_type_sgadvanced ""; cnt_type "Counter type"; cnt_to "Count to value"; operation_sgadvanced ""; operation "Count direction"; start_count "Initial value"; cnt_by_val "Step"; arith_type_sgadvanced ""; arith_type "Output type"; n_bits "Number of bits"; bin_pt "Binary point"; load_pin_sgadvanced ""; load_pin "Provide load port"; rst_sgadvanced ""; rst "Provide synchronous reset port"; en_sgadvanced ""; en "Provide enable port"; explicit_period_sgadvanced ""; explicit_period "Sample period source"; period "Explicit period"; dbl_ovrd_sgadvanced ""; dbl_ovrd "Override with doubles"; use_behavioral_HDL_sgadvanced ""; use_behavioral_HDL "Use behavioral HDL (otherwise use core)"; implementation_sgadvanced ""; implementation "Implement using"; xl_use_area_sgadvanced ""; xl_use_area "Define FPGA area for resource estimation"; xl_area "FPGA area [slices, FFs, BRAMs, LUTs, IOBs, emb. mults, TBUFs]"; use_rpm ""; xlcconfig ""; has_advanced_control ""; sggui_pos ""; block_type ""; block_version ""; sg_icon_stat ""; sg_mask_display ""; sg_list_contents ""; sg_blockgui_xml ""
      mask help: Hardware notes: Free running counters are the least expensive in hardware. A count limited counter is implemented by combining a counter with a comparator.
      mask init: same as med_16a_2x_r113a/buffer1/Counter1
      settings: CopyFcn=xlBlockMoveCallback(gcbh);  DeleteFcn=xlDestroyGui(gcbh);  ModelCloseFcn=xlDestroyGui(gcbh);  PreSaveFcn=xlBlockPreSaveCallback(gcbh);  PostSaveFcn=xlBlockPostSaveCallback(gcbh);  DestroyFcn=xlDestroyGui(gcbh);  OpenFcn=xlOpenGui(gcbh, 'counter_gui.xml', @counterenablement, -1)  CloseFcn=xlDestroyGui(gcbh);  MoveFcn=xlBlockMoveCallback(gcbh);
      mask Help: eval('');xlDoc('-book','sysgen','-topic','Counter');
      mask StyleString: edit,edit,popup(Free Running|Count Limited),edit,edit,popup(Up|Down|Up/Down),edit,edit,edit,popup(Signed  (2's comp)|Unsigned),edit,edit,edit,checkbox,edit,checkbox,edit,checkbox,edit,popup(on|off),edit,edit,checkbox,edit,checkbox,edit,popup(Fabric|DSP48),edit,checkbox,edit,edit,edit,edit,edit,edit,edit,edit,edit,edit,edit
      mask Display:
      | fprintf('','COMMENT: begin icon graphics');
      | patch([0 1 1 0 ],[0 0 1 1 ],[0.77 0.82 0.91]);
      | patch([0.225 0.0625 0.3 0.0625 0.225 0.4875 0.5625 0.6375 0.925 0.7 0.4875 0.3375 0.575 0.3375 0.4875 0.7 0.925 0.6375 0.5625 0.4875 0.225 ],[0.32967 0.401099 0.505495 0.60989 0.681319 0.681319 0.648352 0.681319 0.681319 0.582418 0.675824 0.60989 0.505495 0.401099 0.335165 0.428571 0.32967 0.32967 0.362637 0.32967 0.32967 ],[0.98 0.96 0.92]);
      | plot([0 1 1 0 0 ],[0 0 1 1 0 ]);
      | fprintf('','COMMENT: end icon graphics');
      | fprintf('','COMMENT: begin icon text');
      | color('black');port_label('input',1,'rst');
      | color('black');port_label('input',2,'en');
      | color('black');port_label('output',1,'out');
      | fprintf('','COMMENT: end icon text');
  write_ctrl : Subsystem  mask{N_xeng=N_xeng, N_chans=N_chans}
      mask prompts: N_xeng "Number of X engines"; N_chans "Number of Channels (2^?)"
  xeng_sync_ctr : S-Function  LoadFcn=xlBlockLoadCallback(gcbh);  FunctionName=sysgen  Parameters=ptable_  EnableBusSupport=off  mask(Xilinx Counter Block){infoedit=Hardware notes: Free running counters are the least expensive in hardware.  A count limited counter is implemented by combining a counter with a comparator., cnt_type_sgadvanced=, cnt_type=Count Limited, cnt_to=(N_ants*2^(N_int))-1, operation_sgadvanced=, operation=Up, start_count=0, cnt_by_val=1, arith_type_sgadvanced=, arith_type=Unsigned, n_bits=ceil(log2(N_ants*2^(N_int))), bin_pt=0, load_pin_sgadvanced=, load_pin=off, rst_sgadvanced=, rst=on, en_sgadvanced=, en=off, explicit_period_sgadvanced=, explicit_period=off, period=1, dbl_ovrd_sgadvanced=, dbl_ovrd=off, use_behavioral_HDL_sgadvanced=, use_behavioral_HDL=off, implementation_sgadvanced=, implementation=Fabric, xl_use_area_sgadvanced=, xl_use_area=off, xl_area=[0, 0, 0, 0, 0, 0, 0], use_rpm=on, xlcconfig=, has_advanced_control=0, sggui_pos=368,46,1038,822, block_type=counter, block_version=9.2.01, sg_icon_stat=90,68,1,1,white,blue,0,c59595e6,right,, sg_mask_display=|, sg_list_contents=, sg_blockgui_xml=}
      mask sg_mask_display=|
      | fprintf('','COMMENT: begin icon graphics');
      | patch([0 1 1 0 ],[0 0 1 1 ],[0.77 0.82 0.91]);
      | patch([0.3 0.177778 0.355556 0.177778 0.3 0.5 0.555556 0.611111 0.822222 0.655556 0.5 0.377778 0.544444 0.377778 0.5 0.655556 0.822222 0.611111 0.555556 0.5 0.3 ],[0.102941 0.264706 0.5 0.735294 0.897059 0.897059 0.823529 0.897059 0.897059 0.676471 0.882353 0.720588 0.5 0.279412 0.117647 0.323529 0.102941 0.102941 0.176471 0.102941 0.102941 ],[0.98 0.96 0.92]);
      | plot([0 1 1 0 0 ],[0 0 1 1 0 ]);
      | fprintf('','COMMENT: end icon graphics');
      | fprintf('','COMMENT: begin icon text');
      | color('black');port_label('input',1,'rst');
      | color('black');port_label('output',1,'out');
      | fprintf('','COMMENT: end icon text');
      mask prompts: infoedit ""; cnt_type_sgadvanced ""; cnt_type "Counter type"; cnt_to "Count to value"; operation_sgadvanced ""; operation "Count direction"; start_count "Initial value"; cnt_by_val "Step"; arith_type_sgadvanced ""; arith_type "Output type"; n_bits "Number of bits"; bin_pt "Binary point"; load_pin_sgadvanced ""; load_pin "Provide load port"; rst_sgadvanced ""; rst "Provide synchronous reset port"; en_sgadvanced ""; en "Provide enable port"; explicit_period_sgadvanced ""; explicit_period "Sample period source"; period "Explicit period"; dbl_ovrd_sgadvanced ""; dbl_ovrd "Override with doubles"; use_behavioral_HDL_sgadvanced ""; use_behavioral_HDL "Use behavioral HDL (otherwise use core)"; implementation_sgadvanced ""; implementation "Implement using"; xl_use_area_sgadvanced ""; xl_use_area "Define FPGA area for resource estimation"; xl_area "FPGA area [slices, FFs, BRAMs, LUTs, IOBs, emb. mults, TBUFs]"; use_rpm ""; xlcconfig ""; has_advanced_control ""; sggui_pos ""; block_type ""; block_version ""; sg_icon_stat ""; sg_mask_display ""; sg_list_contents ""; sg_blockgui_xml ""
      mask help: Hardware notes: Free running counters are the least expensive in hardware. A count limited counter is implemented by combining a counter with a comparator.
      mask init: same as med_16a_2x_r113a/buffer1/Counter1
      settings: CopyFcn=xlBlockMoveCallback(gcbh);  DeleteFcn=xlDestroyGui(gcbh);  ModelCloseFcn=xlDestroyGui(gcbh);  PreSaveFcn=xlBlockPreSaveCallback(gcbh);  PostSaveFcn=xlBlockPostSaveCallback(gcbh);  DestroyFcn=xlDestroyGui(gcbh);  OpenFcn=xlOpenGui(gcbh, 'counter_gui.xml', @counterenablement, -1)  CloseFcn=xlDestroyGui(gcbh);  MoveFcn=xlBlockMoveCallback(gcbh);
      mask Help: eval('');xlDoc('-book','sysgen','-topic','Counter');
      mask StyleString: edit,edit,popup(Free Running|Count Limited),edit,edit,popup(Up|Down|Up/Down),edit,edit,edit,popup(Signed  (2's comp)|Unsigned),edit,edit,edit,checkbox,edit,checkbox,edit,checkbox,edit,popup(on|off),edit,edit,checkbox,edit,checkbox,edit,popup(Fabric|DSP48),edit,checkbox,edit,edit,edit,edit,edit,edit,edit,edit,edit,edit,edit
      mask Display:
      | fprintf('','COMMENT: begin icon graphics');
      | patch([0 1 1 0 ],[0 0 1 1 ],[0.77 0.82 0.91]);
      | patch([0.3 0.177778 0.355556 0.177778 0.3 0.5 0.555556 0.611111 0.822222 0.655556 0.5 0.377778 0.544444 0.377778 0.5 0.655556 0.822222 0.611111 0.555556 0.5 0.3 ],[0.102941 0.264706 0.5 0.735294 0.897059 0.897059 0.823529 0.897059 0.897059 0.676471 0.882353 0.720588 0.5 0.279412 0.117647 0.323529 0.102941 0.102941 0.176471 0.102941 0.102941 ],[0.98 0.96 0.92]);
      | plot([0 1 1 0 0 ],[0 0 1 1 0 ]);
      | fprintf('','COMMENT: end icon graphics');
      | fprintf('','COMMENT: begin icon text');
      | color('black');port_label('input',1,'rst');
      | color('black');port_label('output',1,'out');
      | fprintf('','COMMENT: end icon text');
  sync_out : Outport
  dout : Outport  Port=2
  window_vld : Outport  Port=3
  mcnt_out : Outport  Port=4
  wires:
  [read_0] -> Logical10/1
  [read_1] -> Logical8/1
  [mrst] -> Logical10/2, Logical8/2
  Logical10/1 -> Register2/2
  Logical8/1 -> Register3/2
  Logical4/1 -> Logical9/2
  Inverter1/1 -> Logical9/1
  Logical9/1 -> pipeline3/1(d)
  Logical5/1 -> sync_out/1
  Slice4/1 -> Logical5/2
  pipeline3/1(q) -> [read_1]
  pipeline2/1(q) -> [read_0]
  Inverter5/1 -> Delay9/1
  Delay10/1 -> Logical1/1
  Delay9/1 -> Logical/1
  Delay8/1 -> window_vld/1
  pulse_ext/1(out) -> Counter1/2, Logical6/2
  Delay/1 -> Logical5/1, Delay8/1
  mrst/1 -> [mrst]
  Constant1/1 -> rx_buf/2
  window_position/1 -> Relational3/1, Concat/2
  mcnt/1 -> [mcnt]
  eof/1 -> [eof]
  [read_0] -> pulse_ext/1(in), Counter1/1
  ant/1 -> [ant]
  end_window_detect/1(Out1) -> window_position/1
  [ant] -> end_window_detect/2(ant)
  Constant4/1 -> Relational3/2
  Relational3/1 -> Logical3/1
  Logical3/1 -> [block_done]
  Delay4/1 -> Logical3/2
  [eof] -> end_window_detect/1(eof)
  vld/1 -> [vld]
  [vld] -> window_position/2
  [buf_sel] -> Concat/1
  Concat/1 -> [write_addr]
  [vld] -> Delay4/1
  [mrst] -> xeng_sync_ctr/1
  xeng_sync_ctr/1 -> Relational1/1
  Relational1/1 -> [send_window]
  Constant3/1 -> Relational1/2
  Slice3/1 -> Delay3/1
  rx_buf/1 -> Slice3/1, Slice4/1
  Delay3/1 -> dout/1
  [mcnt] -> Slice5/1
  Slice5/1 -> [buf_sel]
  [buf_sel] -> Inverter5/1
  [block_done] -> Logical/2
  [buf_sel] -> Delay10/1
  [block_done] -> Logical1/2
  Logical/1 -> Register2/1, Register2/3, Register4/2
  Logical1/1 -> Register3/1, Register3/3, Register5/2
  Register2/1 -> [buf0_rdy]
  Register3/1 -> [buf1_rdy]
  [send_window] -> Logical2/1
  [buf0_rdy] -> Logical2/2
  [send_window] -> Logical4/1
  [buf1_rdy] -> Logical4/2
  Logical2/1 -> pipeline2/1(d), Inverter1/1
  pulse_ext1/1(out) -> Counter2/2, Logical6/1
  [read_1] -> Counter2/1, pulse_ext1/1(in)
  Counter1/1 -> Concat2/2
  Constant2/1 -> Concat2/1
  Counter2/1 -> Concat3/2
  Constant5/1 -> Concat3/1
  Mux2/1 -> rx_buf/1
  Concat2/1 -> Mux2/2
  Concat3/1 -> Mux2/3
  [read_1] -> pulse_ext2/1(in)
  pulse_ext2/1(out) -> Mux2/1
  Logical6/1 -> rx_buf/3, Delay/1
  Logical7/1 -> Delay5/1
  [mcnt] -> Delay1/1
  Delay1/1 -> Register4/1
  Register4/1 -> [buf0_mcnt]
  [mcnt] -> Delay2/1
  Delay2/1 -> Register5/1
  Register5/1 -> [buf1_mcnt]
  Register6/1 -> Logical7/1
  Register7/1 -> Logical7/2
  [buf0_mcnt] -> Register6/1
  Delay5/1 -> mcnt_out/1
  [buf1_mcnt] -> Register7/1
  pulse_ext3/1(out) -> Inverter3/1
  Inverter3/1 -> Register6/2
  [read_0] -> pulse_ext3/1(in), Delay7/1
  [read_1] -> pulse_ext4/1(in), Delay6/1
  pulse_ext4/1(out) -> Inverter4/1
  Inverter4/1 -> Register7/2
  Delay6/1 -> Register7/3
  Delay7/1 -> Register6/3
  write_ctrl/2(dout) -> rx_buf/5
  write_ctrl/3(we) -> rx_buf/6
  write_ctrl/1(addr_out) -> rx_buf/4
  [write_addr] -> write_ctrl/1(addr_in)
  [mcnt] -> write_ctrl/2(mcnt)
  [vld] -> write_ctrl/3(vld)
  din/1 -> write_ctrl/4(din)
